simulink model "cddDcmApp"  (R2022b)
settings: Solver=FixedStepDiscrete  StartTime=0.0  StopTime=10.0  FixedStep=0.02  RelTol=1e-3
undefined symbols (not defined in the file or in its folder): CCPFaultType, DcChlType, HFAChlType, IcuChannelType, MtrRoutStaEnum, P_SignalPartType, P_Std_ReturnType, PowerChlType, SrsAdChlType, VGAChlType
(part 6 of 13)

== system cddDcmApp/DataServices_DID_980A_2_Front_ventfloor_actuator_positionfront_vent_actuator_position/DataServices_DID_980A_2_Front_ventfloor_actuator_positionfront_vent_actuator_position_FreezeCurrentState_sys ==
  function : TriggerPort  TriggerType=function-call  IsSimulinkFunction=on  FunctionName=DataServices_DID_980A_2_Front_ventfloor_actuator_positionfront_vent_actuator_position_FreezeCurrentState  VariantControl=(inherit)
  Ground_1 : Ground
  Ground_2 : Ground
  Terminator_1 : Terminator
  OpStatus : ArgIn  OutDataTypeStr=Enum: Dcm_OpStatusType  ArgumentName=OpStatus
  ErrorCode : ArgOut  OutDataTypeStr=Enum: Dcm_NegativeResponseCodeType  ArgumentName=ErrorCode
  ERR : ArgOut  Port=2  OutDataTypeStr=Std_ReturnType  ArgumentName=ERR
  wires:
  OpStatus/1 -> Terminator_1/1
  Ground_1/1 -> ErrorCode/1
  Ground_2/1 -> ERR/1

== system cddDcmApp/DataServices_DID_980A_2_Front_ventfloor_actuator_positionfront_vent_actuator_position/DataServices_DID_980A_2_Front_ventfloor_actuator_positionfront_vent_actuator_position_ReadData_sys ==
  function : TriggerPort  TriggerType=function-call  IsSimulinkFunction=on  FunctionName=DataServices_DID_980A_2_Front_ventfloor_actuator_positionfront_vent_actuator_position_ReadData  VariantControl=(inherit)
  Ground_1 : Ground
  Ground_2 : Ground
  Terminator_1 : Terminator
  OpStatus : ArgIn  OutDataTypeStr=Enum: Dcm_OpStatusType  ArgumentName=OpStatus
  Data : ArgOut  OutDataTypeStr=uint8  ArgumentName=Data
  ERR : ArgOut  Port=2  OutDataTypeStr=Std_ReturnType  ArgumentName=ERR
  wires:
  OpStatus/1 -> Terminator_1/1
  Ground_1/1 -> Data/1
  Ground_2/1 -> ERR/1

== system cddDcmApp/DataServices_DID_980A_2_Front_ventfloor_actuator_positionfront_vent_actuator_position/DataServices_DID_980A_2_Front_ventfloor_actuator_positionfront_vent_actuator_position_ReturnControlToECU_sys ==
  function : TriggerPort  TriggerType=function-call  IsSimulinkFunction=on  FunctionName=DataServices_DID_980A_2_Front_ventfloor_actuator_positionfront_vent_actuator_position_ReturnControlToECU  VariantControl=(inherit)
  Ground_1 : Ground
  Ground_2 : Ground
  ErrorCode : ArgOut  OutDataTypeStr=Enum: Dcm_NegativeResponseCodeType  ArgumentName=ErrorCode
  ERR : ArgOut  Port=2  OutDataTypeStr=Std_ReturnType  ArgumentName=ERR
  wires:
  Ground_1/1 -> ErrorCode/1
  Ground_2/1 -> ERR/1

== system cddDcmApp/DataServices_DID_980A_2_Front_ventfloor_actuator_positionfront_vent_actuator_position/DataServices_DID_980A_2_Front_ventfloor_actuator_positionfront_vent_actuator_position_ShortTermAdjustment_sys ==
  function : TriggerPort  TriggerType=function-call  IsSimulinkFunction=on  FunctionName=DataServices_DID_980A_2_Front_ventfloor_actuator_positionfront_vent_actuator_position_ShortTermAdjustment  VariantControl=(inherit)
  Ground_1 : Ground
  Ground_2 : Ground
  Terminator_1 : Terminator
  Terminator_2 : Terminator
  ControlStateInfo : ArgIn  OutDataTypeStr=uint8  ArgumentName=ControlStateInfo
  OpStatus : ArgIn  Port=2  OutDataTypeStr=Enum: Dcm_OpStatusType  ArgumentName=OpStatus
  ErrorCode : ArgOut  OutDataTypeStr=Enum: Dcm_NegativeResponseCodeType  ArgumentName=ErrorCode
  ERR : ArgOut  Port=2  OutDataTypeStr=Std_ReturnType  ArgumentName=ERR
  wires:
  ControlStateInfo/1 -> Terminator_1/1
  OpStatus/1 -> Terminator_2/1
  Ground_1/1 -> ErrorCode/1
  Ground_2/1 -> ERR/1

== system cddDcmApp/DataServices_DID_980A_DID_980A_1_Deforst_actuator_position/DataServices_DID_980A_DID_980A_1_Deforst_actuator_position_FreezeCurrentState_sys ==
  function : TriggerPort  TriggerType=function-call  IsSimulinkFunction=on  FunctionName=DataServices_DID_980A_DID_980A_1_Deforst_actuator_position_FreezeCurrentState  VariantControl=(inherit)
  Ground_1 : Ground
  Ground_2 : Ground
  Terminator_1 : Terminator
  OpStatus : ArgIn  OutDataTypeStr=Enum: Dcm_OpStatusType  ArgumentName=OpStatus
  ErrorCode : ArgOut  OutDataTypeStr=Enum: Dcm_NegativeResponseCodeType  ArgumentName=ErrorCode
  ERR : ArgOut  Port=2  OutDataTypeStr=Std_ReturnType  ArgumentName=ERR
  wires:
  OpStatus/1 -> Terminator_1/1
  Ground_1/1 -> ErrorCode/1
  Ground_2/1 -> ERR/1

== system cddDcmApp/DataServices_DID_980A_DID_980A_1_Deforst_actuator_position/DataServices_DID_980A_DID_980A_1_Deforst_actuator_position_ReadData_sys ==
  function : TriggerPort  TriggerType=function-call  IsSimulinkFunction=on  FunctionName=DataServices_DID_980A_DID_980A_1_Deforst_actuator_position_ReadData  VariantControl=(inherit)
  Ground_1 : Ground
  Ground_2 : Ground
  Terminator_1 : Terminator
  OpStatus : ArgIn  OutDataTypeStr=Enum: Dcm_OpStatusType  ArgumentName=OpStatus
  Data : ArgOut  OutDataTypeStr=uint8  ArgumentName=Data
  ERR : ArgOut  Port=2  OutDataTypeStr=Std_ReturnType  ArgumentName=ERR
  wires:
  OpStatus/1 -> Terminator_1/1
  Ground_1/1 -> Data/1
  Ground_2/1 -> ERR/1

== system cddDcmApp/DataServices_DID_980A_DID_980A_1_Deforst_actuator_position/DataServices_DID_980A_DID_980A_1_Deforst_actuator_position_ReturnControlToECU_sys ==
  function : TriggerPort  TriggerType=function-call  IsSimulinkFunction=on  FunctionName=DataServices_DID_980A_DID_980A_1_Deforst_actuator_position_ReturnControlToECU  VariantControl=(inherit)
  Ground_1 : Ground
  Ground_2 : Ground
  ErrorCode : ArgOut  OutDataTypeStr=Enum: Dcm_NegativeResponseCodeType  ArgumentName=ErrorCode
  ERR : ArgOut  Port=2  OutDataTypeStr=Std_ReturnType  ArgumentName=ERR
  wires:
  Ground_1/1 -> ErrorCode/1
  Ground_2/1 -> ERR/1

== system cddDcmApp/DataServices_DID_980A_DID_980A_1_Deforst_actuator_position/DataServices_DID_980A_DID_980A_1_Deforst_actuator_position_ShortTermAdjustment_sys ==
  function : TriggerPort  TriggerType=function-call  IsSimulinkFunction=on  FunctionName=DataServices_DID_980A_DID_980A_1_Deforst_actuator_position_ShortTermAdjustment  VariantControl=(inherit)
  Ground_1 : Ground
  Ground_2 : Ground
  Terminator_1 : Terminator
  Terminator_2 : Terminator
  ControlStateInfo : ArgIn  OutDataTypeStr=uint8  ArgumentName=ControlStateInfo
  OpStatus : ArgIn  Port=2  OutDataTypeStr=Enum: Dcm_OpStatusType  ArgumentName=OpStatus
  ErrorCode : ArgOut  OutDataTypeStr=Enum: Dcm_NegativeResponseCodeType  ArgumentName=ErrorCode
  ERR : ArgOut  Port=2  OutDataTypeStr=Std_ReturnType  ArgumentName=ERR
  wires:
  ControlStateInfo/1 -> Terminator_1/1
  OpStatus/1 -> Terminator_2/1
  Ground_1/1 -> ErrorCode/1
  Ground_2/1 -> ERR/1

== system cddDcmApp/DataServices_DID_980A_DID_980A_3_Front_floor_actuator_positionposition/DataServices_DID_980A_DID_980A_3_Front_floor_actuator_positionposition_FreezeCurrentState_sys ==
  function : TriggerPort  TriggerType=function-call  IsSimulinkFunction=on  FunctionName=DataServices_DID_980A_DID_980A_3_Front_floor_actuator_positionposition_FreezeCurrentState  VariantControl=(inherit)
  Ground_1 : Ground
  Ground_2 : Ground
  Terminator_1 : Terminator
  OpStatus : ArgIn  OutDataTypeStr=Enum: Dcm_OpStatusType  ArgumentName=OpStatus
  ErrorCode : ArgOut  OutDataTypeStr=Enum: Dcm_NegativeResponseCodeType  ArgumentName=ErrorCode
  ERR : ArgOut  Port=2  OutDataTypeStr=Std_ReturnType  ArgumentName=ERR
  wires:
  OpStatus/1 -> Terminator_1/1
  Ground_1/1 -> ErrorCode/1
  Ground_2/1 -> ERR/1

== system cddDcmApp/DataServices_DID_980A_DID_980A_3_Front_floor_actuator_positionposition/DataServices_DID_980A_DID_980A_3_Front_floor_actuator_positionposition_ReadData_sys ==
  function : TriggerPort  TriggerType=function-call  IsSimulinkFunction=on  FunctionName=DataServices_DID_980A_DID_980A_3_Front_floor_actuator_positionposition_ReadData  VariantControl=(inherit)
  Ground_1 : Ground
  Ground_2 : Ground
  Terminator_1 : Terminator
  OpStatus : ArgIn  OutDataTypeStr=Enum: Dcm_OpStatusType  ArgumentName=OpStatus
  Data : ArgOut  OutDataTypeStr=uint8  ArgumentName=Data
  ERR : ArgOut  Port=2  OutDataTypeStr=Std_ReturnType  ArgumentName=ERR
  wires:
  OpStatus/1 -> Terminator_1/1
  Ground_1/1 -> Data/1
  Ground_2/1 -> ERR/1

== system cddDcmApp/DataServices_DID_980A_DID_980A_3_Front_floor_actuator_positionposition/DataServices_DID_980A_DID_980A_3_Front_floor_actuator_positionposition_ReturnControlToECU_sys ==
  function : TriggerPort  TriggerType=function-call  IsSimulinkFunction=on  FunctionName=DataServices_DID_980A_DID_980A_3_Front_floor_actuator_positionposition_ReturnControlToECU  VariantControl=(inherit)
  Ground_1 : Ground
  Ground_2 : Ground
  ErrorCode : ArgOut  OutDataTypeStr=Enum: Dcm_NegativeResponseCodeType  ArgumentName=ErrorCode
  ERR : ArgOut  Port=2  OutDataTypeStr=Std_ReturnType  ArgumentName=ERR
  wires:
  Ground_1/1 -> ErrorCode/1
  Ground_2/1 -> ERR/1

== system cddDcmApp/DataServices_DID_980A_DID_980A_3_Front_floor_actuator_positionposition/DataServices_DID_980A_DID_980A_3_Front_floor_actuator_positionposition_ShortTermAdjustment_sys ==
  function : TriggerPort  TriggerType=function-call  IsSimulinkFunction=on  FunctionName=DataServices_DID_980A_DID_980A_3_Front_floor_actuator_positionposition_ShortTermAdjustment  VariantControl=(inherit)
  Ground_1 : Ground
  Ground_2 : Ground
  Terminator_1 : Terminator
  Terminator_2 : Terminator
  ControlStateInfo : ArgIn  OutDataTypeStr=uint8  ArgumentName=ControlStateInfo
  OpStatus : ArgIn  Port=2  OutDataTypeStr=Enum: Dcm_OpStatusType  ArgumentName=OpStatus
  ErrorCode : ArgOut  OutDataTypeStr=Enum: Dcm_NegativeResponseCodeType  ArgumentName=ErrorCode
  ERR : ArgOut  Port=2  OutDataTypeStr=Std_ReturnType  ArgumentName=ERR
  wires:
  ControlStateInfo/1 -> Terminator_1/1
  OpStatus/1 -> Terminator_2/1
  Ground_1/1 -> ErrorCode/1
  Ground_2/1 -> ERR/1

== system cddDcmApp/DataServices_DID_980A_DID_980A_4_Second_row_ventventfloor_actuator_position/DataServices_DID_980A_DID_980A_4_Second_row_ventventfloor_actuator_position_FreezeCurrentState_sys ==
  function : TriggerPort  TriggerType=function-call  IsSimulinkFunction=on  FunctionName=DataServices_DID_980A_DID_980A_4_Second_row_ventventfloor_actuator_position_FreezeCurrentState  VariantControl=(inherit)
  Ground_1 : Ground
  Ground_2 : Ground
  Terminator_1 : Terminator
  OpStatus : ArgIn  OutDataTypeStr=Enum: Dcm_OpStatusType  ArgumentName=OpStatus
  ErrorCode : ArgOut  OutDataTypeStr=Enum: Dcm_NegativeResponseCodeType  ArgumentName=ErrorCode
  ERR : ArgOut  Port=2  OutDataTypeStr=Std_ReturnType  ArgumentName=ERR
  wires:
  OpStatus/1 -> Terminator_1/1
  Ground_1/1 -> ErrorCode/1
  Ground_2/1 -> ERR/1

== system cddDcmApp/DataServices_DID_980A_DID_980A_4_Second_row_ventventfloor_actuator_position/DataServices_DID_980A_DID_980A_4_Second_row_ventventfloor_actuator_position_ReadData_sys ==
  function : TriggerPort  TriggerType=function-call  IsSimulinkFunction=on  FunctionName=DataServices_DID_980A_DID_980A_4_Second_row_ventventfloor_actuator_position_ReadData  VariantControl=(inherit)
  Ground_1 : Ground
  Ground_2 : Ground
  Terminator_1 : Terminator
  OpStatus : ArgIn  OutDataTypeStr=Enum: Dcm_OpStatusType  ArgumentName=OpStatus
  Data : ArgOut  OutDataTypeStr=uint8  ArgumentName=Data
  ERR : ArgOut  Port=2  OutDataTypeStr=Std_ReturnType  ArgumentName=ERR
  wires:
  OpStatus/1 -> Terminator_1/1
  Ground_1/1 -> Data/1
  Ground_2/1 -> ERR/1

== system cddDcmApp/DataServices_DID_980A_DID_980A_4_Second_row_ventventfloor_actuator_position/DataServices_DID_980A_DID_980A_4_Second_row_ventventfloor_actuator_position_ReturnControlToECU_sys ==
  function : TriggerPort  TriggerType=function-call  IsSimulinkFunction=on  FunctionName=DataServices_DID_980A_DID_980A_4_Second_row_ventventfloor_actuator_position_ReturnControlToECU  VariantControl=(inherit)
  Ground_1 : Ground
  Ground_2 : Ground
  ErrorCode : ArgOut  OutDataTypeStr=Enum: Dcm_NegativeResponseCodeType  ArgumentName=ErrorCode
  ERR : ArgOut  Port=2  OutDataTypeStr=Std_ReturnType  ArgumentName=ERR
  wires:
  Ground_1/1 -> ErrorCode/1
  Ground_2/1 -> ERR/1

== system cddDcmApp/DataServices_DID_980A_DID_980A_4_Second_row_ventventfloor_actuator_position/DataServices_DID_980A_DID_980A_4_Second_row_ventventfloor_actuator_position_ShortTermAdjustment_sys ==
  function : TriggerPort  TriggerType=function-call  IsSimulinkFunction=on  FunctionName=DataServices_DID_980A_DID_980A_4_Second_row_ventventfloor_actuator_position_ShortTermAdjustment  VariantControl=(inherit)
  Ground_1 : Ground
  Ground_2 : Ground
  Terminator_1 : Terminator
  Terminator_2 : Terminator
  ControlStateInfo : ArgIn  OutDataTypeStr=uint8  ArgumentName=ControlStateInfo
  OpStatus : ArgIn  Port=2  OutDataTypeStr=Enum: Dcm_OpStatusType  ArgumentName=OpStatus
  ErrorCode : ArgOut  OutDataTypeStr=Enum: Dcm_NegativeResponseCodeType  ArgumentName=ErrorCode
  ERR : ArgOut  Port=2  OutDataTypeStr=Std_ReturnType  ArgumentName=ERR
  wires:
  ControlStateInfo/1 -> Terminator_1/1
  OpStatus/1 -> Terminator_2/1
  Ground_1/1 -> ErrorCode/1
  Ground_2/1 -> ERR/1

== system cddDcmApp/DataServices_DID_980B_DID_980B_10_VGA7Passenger_Right_X_actuator_positon/DataServices_DID_980B_DID_980B_10_VGA7Passenger_Right_X_actuator_positon_FreezeCurrentState_sys ==
  function : TriggerPort  TriggerType=function-call  IsSimulinkFunction=on  FunctionName=DataServices_DID_980B_DID_980B_10_VGA7Passenger_Right_X_actuator_positon_FreezeCurrentState  VariantControl=(inherit)
  Ground_1 : Ground
  Ground_2 : Ground
  Terminator_1 : Terminator
  OpStatus : ArgIn  OutDataTypeStr=Enum: Dcm_OpStatusType  ArgumentName=OpStatus
  ErrorCode : ArgOut  OutDataTypeStr=Enum: Dcm_NegativeResponseCodeType  ArgumentName=ErrorCode
  ERR : ArgOut  Port=2  OutDataTypeStr=Std_ReturnType  ArgumentName=ERR
  wires:
  OpStatus/1 -> Terminator_1/1
  Ground_1/1 -> ErrorCode/1
  Ground_2/1 -> ERR/1

== system cddDcmApp/DataServices_DID_980B_DID_980B_10_VGA7Passenger_Right_X_actuator_positon/DataServices_DID_980B_DID_980B_10_VGA7Passenger_Right_X_actuator_positon_ReadData_sys ==
  function : TriggerPort  TriggerType=function-call  IsSimulinkFunction=on  FunctionName=DataServices_DID_980B_DID_980B_10_VGA7Passenger_Right_X_actuator_positon_ReadData  VariantControl=(inherit)
  Ground_1 : Ground
  Ground_2 : Ground
  Terminator_1 : Terminator
  OpStatus : ArgIn  OutDataTypeStr=Enum: Dcm_OpStatusType  ArgumentName=OpStatus
  Data : ArgOut  OutDataTypeStr=uint8  ArgumentName=Data
  ERR : ArgOut  Port=2  OutDataTypeStr=Std_ReturnType  ArgumentName=ERR
  wires:
  OpStatus/1 -> Terminator_1/1
  Ground_1/1 -> Data/1
  Ground_2/1 -> ERR/1

== system cddDcmApp/DataServices_DID_980B_DID_980B_10_VGA7Passenger_Right_X_actuator_positon/DataServices_DID_980B_DID_980B_10_VGA7Passenger_Right_X_actuator_positon_ReturnControlToECU_sys ==
  function : TriggerPort  TriggerType=function-call  IsSimulinkFunction=on  FunctionName=DataServices_DID_980B_DID_980B_10_VGA7Passenger_Right_X_actuator_positon_ReturnControlToECU  VariantControl=(inherit)
  Ground_1 : Ground
  Ground_2 : Ground
  ErrorCode : ArgOut  OutDataTypeStr=Enum: Dcm_NegativeResponseCodeType  ArgumentName=ErrorCode
  ERR : ArgOut  Port=2  OutDataTypeStr=Std_ReturnType  ArgumentName=ERR
  wires:
  Ground_1/1 -> ErrorCode/1
  Ground_2/1 -> ERR/1

== system cddDcmApp/DataServices_DID_980B_DID_980B_10_VGA7Passenger_Right_X_actuator_positon/DataServices_DID_980B_DID_980B_10_VGA7Passenger_Right_X_actuator_positon_ShortTermAdjustment_sys ==
  function : TriggerPort  TriggerType=function-call  IsSimulinkFunction=on  FunctionName=DataServices_DID_980B_DID_980B_10_VGA7Passenger_Right_X_actuator_positon_ShortTermAdjustment  VariantControl=(inherit)
  Ground_1 : Ground
  Ground_2 : Ground
  Terminator_1 : Terminator
  Terminator_2 : Terminator
  ControlStateInfo : ArgIn  OutDataTypeStr=uint8  ArgumentName=ControlStateInfo
  OpStatus : ArgIn  Port=2  OutDataTypeStr=Enum: Dcm_OpStatusType  ArgumentName=OpStatus
  ErrorCode : ArgOut  OutDataTypeStr=Enum: Dcm_NegativeResponseCodeType  ArgumentName=ErrorCode
  ERR : ArgOut  Port=2  OutDataTypeStr=Std_ReturnType  ArgumentName=ERR
  wires:
  ControlStateInfo/1 -> Terminator_1/1
  OpStatus/1 -> Terminator_2/1
  Ground_1/1 -> ErrorCode/1
  Ground_2/1 -> ERR/1

== system cddDcmApp/DataServices_DID_980B_DID_980B_11_VGA8Passenger_Right_Y_actuator_positon/DataServices_DID_980B_DID_980B_11_VGA8Passenger_Right_Y_actuator_positon_FreezeCurrentState_sys ==
  function : TriggerPort  TriggerType=function-call  IsSimulinkFunction=on  FunctionName=DataServices_DID_980B_DID_980B_11_VGA8Passenger_Right_Y_actuator_positon_FreezeCurrentState  VariantControl=(inherit)
  Ground_1 : Ground
  Ground_2 : Ground
  Terminator_1 : Terminator
  OpStatus : ArgIn  OutDataTypeStr=Enum: Dcm_OpStatusType  ArgumentName=OpStatus
  ErrorCode : ArgOut  OutDataTypeStr=Enum: Dcm_NegativeResponseCodeType  ArgumentName=ErrorCode
  ERR : ArgOut  Port=2  OutDataTypeStr=Std_ReturnType  ArgumentName=ERR
  wires:
  OpStatus/1 -> Terminator_1/1
  Ground_1/1 -> ErrorCode/1
  Ground_2/1 -> ERR/1

== system cddDcmApp/DataServices_DID_980B_DID_980B_11_VGA8Passenger_Right_Y_actuator_positon/DataServices_DID_980B_DID_980B_11_VGA8Passenger_Right_Y_actuator_positon_ReadData_sys ==
  function : TriggerPort  TriggerType=function-call  IsSimulinkFunction=on  FunctionName=DataServices_DID_980B_DID_980B_11_VGA8Passenger_Right_Y_actuator_positon_ReadData  VariantControl=(inherit)
  Ground_1 : Ground
  Ground_2 : Ground
  Terminator_1 : Terminator
  OpStatus : ArgIn  OutDataTypeStr=Enum: Dcm_OpStatusType  ArgumentName=OpStatus
  Data : ArgOut  OutDataTypeStr=uint8  ArgumentName=Data
  ERR : ArgOut  Port=2  OutDataTypeStr=Std_ReturnType  ArgumentName=ERR
  wires:
  OpStatus/1 -> Terminator_1/1
  Ground_1/1 -> Data/1
  Ground_2/1 -> ERR/1

== system cddDcmApp/DataServices_DID_980B_DID_980B_11_VGA8Passenger_Right_Y_actuator_positon/DataServices_DID_980B_DID_980B_11_VGA8Passenger_Right_Y_actuator_positon_ReturnControlToECU_sys ==
  function : TriggerPort  TriggerType=function-call  IsSimulinkFunction=on  FunctionName=DataServices_DID_980B_DID_980B_11_VGA8Passenger_Right_Y_actuator_positon_ReturnControlToECU  VariantControl=(inherit)
  Ground_1 : Ground
  Ground_2 : Ground
  ErrorCode : ArgOut  OutDataTypeStr=Enum: Dcm_NegativeResponseCodeType  ArgumentName=ErrorCode
  ERR : ArgOut  Port=2  OutDataTypeStr=Std_ReturnType  ArgumentName=ERR
  wires:
  Ground_1/1 -> ErrorCode/1
  Ground_2/1 -> ERR/1

== system cddDcmApp/DataServices_DID_980B_DID_980B_11_VGA8Passenger_Right_Y_actuator_positon/DataServices_DID_980B_DID_980B_11_VGA8Passenger_Right_Y_actuator_positon_ShortTermAdjustment_sys ==
  function : TriggerPort  TriggerType=function-call  IsSimulinkFunction=on  FunctionName=DataServices_DID_980B_DID_980B_11_VGA8Passenger_Right_Y_actuator_positon_ShortTermAdjustment  VariantControl=(inherit)
  Ground_1 : Ground
  Ground_2 : Ground
  Terminator_1 : Terminator
  Terminator_2 : Terminator
  ControlStateInfo : ArgIn  OutDataTypeStr=uint8  ArgumentName=ControlStateInfo
  OpStatus : ArgIn  Port=2  OutDataTypeStr=Enum: Dcm_OpStatusType  ArgumentName=OpStatus
  ErrorCode : ArgOut  OutDataTypeStr=Enum: Dcm_NegativeResponseCodeType  ArgumentName=ErrorCode
  ERR : ArgOut  Port=2  OutDataTypeStr=Std_ReturnType  ArgumentName=ERR
  wires:
  ControlStateInfo/1 -> Terminator_1/1
  OpStatus/1 -> Terminator_2/1
  Ground_1/1 -> ErrorCode/1
  Ground_2/1 -> ERR/1

== system cddDcmApp/DataServices_DID_980B_DID_980B_1_second_row_floor_actuator_position/DataServices_DID_980B_DID_980B_1_second_row_floor_actuator_position_FreezeCurrentState_sys ==
  function : TriggerPort  TriggerType=function-call  IsSimulinkFunction=on  FunctionName=DataServices_DID_980B_DID_980B_1_second_row_floor_actuator_position_FreezeCurrentState  VariantControl=(inherit)
  Ground_1 : Ground
  Ground_2 : Ground
  Terminator_1 : Terminator
  OpStatus : ArgIn  OutDataTypeStr=Enum: Dcm_OpStatusType  ArgumentName=OpStatus
  ErrorCode : ArgOut  OutDataTypeStr=Enum: Dcm_NegativeResponseCodeType  ArgumentName=ErrorCode
  ERR : ArgOut  Port=2  OutDataTypeStr=Std_ReturnType  ArgumentName=ERR
  wires:
  OpStatus/1 -> Terminator_1/1
  Ground_1/1 -> ErrorCode/1
  Ground_2/1 -> ERR/1

== system cddDcmApp/DataServices_DID_980B_DID_980B_1_second_row_floor_actuator_position/DataServices_DID_980B_DID_980B_1_second_row_floor_actuator_position_ReadData_sys ==
  function : TriggerPort  TriggerType=function-call  IsSimulinkFunction=on  FunctionName=DataServices_DID_980B_DID_980B_1_second_row_floor_actuator_position_ReadData  VariantControl=(inherit)
  Ground_1 : Ground
  Ground_2 : Ground
  Terminator_1 : Terminator
  OpStatus : ArgIn  OutDataTypeStr=Enum: Dcm_OpStatusType  ArgumentName=OpStatus
  Data : ArgOut  OutDataTypeStr=uint8  ArgumentName=Data
  ERR : ArgOut  Port=2  OutDataTypeStr=Std_ReturnType  ArgumentName=ERR
  wires:
  OpStatus/1 -> Terminator_1/1
  Ground_1/1 -> Data/1
  Ground_2/1 -> ERR/1

== system cddDcmApp/DataServices_DID_980B_DID_980B_1_second_row_floor_actuator_position/DataServices_DID_980B_DID_980B_1_second_row_floor_actuator_position_ReturnControlToECU_sys ==
  function : TriggerPort  TriggerType=function-call  IsSimulinkFunction=on  FunctionName=DataServices_DID_980B_DID_980B_1_second_row_floor_actuator_position_ReturnControlToECU  VariantControl=(inherit)
  Ground_1 : Ground
  Ground_2 : Ground
  ErrorCode : ArgOut  OutDataTypeStr=Enum: Dcm_NegativeResponseCodeType  ArgumentName=ErrorCode
  ERR : ArgOut  Port=2  OutDataTypeStr=Std_ReturnType  ArgumentName=ERR
  wires:
  Ground_1/1 -> ErrorCode/1
  Ground_2/1 -> ERR/1

== system cddDcmApp/DataServices_DID_980B_DID_980B_1_second_row_floor_actuator_position/DataServices_DID_980B_DID_980B_1_second_row_floor_actuator_position_ShortTermAdjustment_sys ==
  function : TriggerPort  TriggerType=function-call  IsSimulinkFunction=on  FunctionName=DataServices_DID_980B_DID_980B_1_second_row_floor_actuator_position_ShortTermAdjustment  VariantControl=(inherit)
  Ground_1 : Ground
  Ground_2 : Ground
  Terminator_1 : Terminator
  Terminator_2 : Terminator
  ControlStateInfo : ArgIn  OutDataTypeStr=uint8  ArgumentName=ControlStateInfo
  OpStatus : ArgIn  Port=2  OutDataTypeStr=Enum: Dcm_OpStatusType  ArgumentName=OpStatus
  ErrorCode : ArgOut  OutDataTypeStr=Enum: Dcm_NegativeResponseCodeType  ArgumentName=ErrorCode
  ERR : ArgOut  Port=2  OutDataTypeStr=Std_ReturnType  ArgumentName=ERR
  wires:
  ControlStateInfo/1 -> Terminator_1/1
  OpStatus/1 -> Terminator_2/1
  Ground_1/1 -> ErrorCode/1
  Ground_2/1 -> ERR/1

== system cddDcmApp/DataServices_DID_980B_DID_980B_2_Rec_actuator_position/DataServices_DID_980B_DID_980B_2_Rec_actuator_position_FreezeCurrentState_sys ==
  function : TriggerPort  TriggerType=function-call  IsSimulinkFunction=on  FunctionName=DataServices_DID_980B_DID_980B_2_Rec_actuator_position_FreezeCurrentState  VariantControl=(inherit)
  Ground_1 : Ground
  Ground_2 : Ground
  Terminator_1 : Terminator
  OpStatus : ArgIn  OutDataTypeStr=Enum: Dcm_OpStatusType  ArgumentName=OpStatus
  ErrorCode : ArgOut  OutDataTypeStr=Enum: Dcm_NegativeResponseCodeType  ArgumentName=ErrorCode
  ERR : ArgOut  Port=2  OutDataTypeStr=Std_ReturnType  ArgumentName=ERR
  wires:
  OpStatus/1 -> Terminator_1/1
  Ground_1/1 -> ErrorCode/1
  Ground_2/1 -> ERR/1

== system cddDcmApp/DataServices_DID_980B_DID_980B_2_Rec_actuator_position/DataServices_DID_980B_DID_980B_2_Rec_actuator_position_ReadData_sys ==
  function : TriggerPort  TriggerType=function-call  IsSimulinkFunction=on  FunctionName=DataServices_DID_980B_DID_980B_2_Rec_actuator_position_ReadData  VariantControl=(inherit)
  Ground_1 : Ground
  Ground_2 : Ground
  Terminator_1 : Terminator
  OpStatus : ArgIn  OutDataTypeStr=Enum: Dcm_OpStatusType  ArgumentName=OpStatus
  Data : ArgOut  OutDataTypeStr=uint8  ArgumentName=Data
  ERR : ArgOut  Port=2  OutDataTypeStr=Std_ReturnType  ArgumentName=ERR
  wires:
  OpStatus/1 -> Terminator_1/1
  Ground_1/1 -> Data/1
  Ground_2/1 -> ERR/1

== system cddDcmApp/DataServices_DID_980B_DID_980B_2_Rec_actuator_position/DataServices_DID_980B_DID_980B_2_Rec_actuator_position_ReturnControlToECU_sys ==
  function : TriggerPort  TriggerType=function-call  IsSimulinkFunction=on  FunctionName=DataServices_DID_980B_DID_980B_2_Rec_actuator_position_ReturnControlToECU  VariantControl=(inherit)
  Ground_1 : Ground
  Ground_2 : Ground
  ErrorCode : ArgOut  OutDataTypeStr=Enum: Dcm_NegativeResponseCodeType  ArgumentName=ErrorCode
  ERR : ArgOut  Port=2  OutDataTypeStr=Std_ReturnType  ArgumentName=ERR
  wires:
  Ground_1/1 -> ErrorCode/1
  Ground_2/1 -> ERR/1

== system cddDcmApp/DataServices_DID_980B_DID_980B_2_Rec_actuator_position/DataServices_DID_980B_DID_980B_2_Rec_actuator_position_ShortTermAdjustment_sys ==
  function : TriggerPort  TriggerType=function-call  IsSimulinkFunction=on  FunctionName=DataServices_DID_980B_DID_980B_2_Rec_actuator_position_ShortTermAdjustment  VariantControl=(inherit)
  Ground_1 : Ground
  Ground_2 : Ground
  Terminator_1 : Terminator
  Terminator_2 : Terminator
  ControlStateInfo : ArgIn  OutDataTypeStr=uint8  ArgumentName=ControlStateInfo
  OpStatus : ArgIn  Port=2  OutDataTypeStr=Enum: Dcm_OpStatusType  ArgumentName=OpStatus
  ErrorCode : ArgOut  OutDataTypeStr=Enum: Dcm_NegativeResponseCodeType  ArgumentName=ErrorCode
  ERR : ArgOut  Port=2  OutDataTypeStr=Std_ReturnType  ArgumentName=ERR
  wires:
  ControlStateInfo/1 -> Terminator_1/1
  OpStatus/1 -> Terminator_2/1
  Ground_1/1 -> ErrorCode/1
  Ground_2/1 -> ERR/1

== system cddDcmApp/DataServices_DID_980B_DID_980B_3_Osa_actuator_position/DataServices_DID_980B_DID_980B_3_Osa_actuator_position_FreezeCurrentState_sys ==
  function : TriggerPort  TriggerType=function-call  IsSimulinkFunction=on  FunctionName=DataServices_DID_980B_DID_980B_3_Osa_actuator_position_FreezeCurrentState  VariantControl=(inherit)
  Ground_1 : Ground
  Ground_2 : Ground
  Terminator_1 : Terminator
  OpStatus : ArgIn  OutDataTypeStr=Enum: Dcm_OpStatusType  ArgumentName=OpStatus
  ErrorCode : ArgOut  OutDataTypeStr=Enum: Dcm_NegativeResponseCodeType  ArgumentName=ErrorCode
  ERR : ArgOut  Port=2  OutDataTypeStr=Std_ReturnType  ArgumentName=ERR
  wires:
  OpStatus/1 -> Terminator_1/1
  Ground_1/1 -> ErrorCode/1
  Ground_2/1 -> ERR/1

== system cddDcmApp/DataServices_DID_980B_DID_980B_3_Osa_actuator_position/DataServices_DID_980B_DID_980B_3_Osa_actuator_position_ReadData_sys ==
  function : TriggerPort  TriggerType=function-call  IsSimulinkFunction=on  FunctionName=DataServices_DID_980B_DID_980B_3_Osa_actuator_position_ReadData  VariantControl=(inherit)
  Ground_1 : Ground
  Ground_2 : Ground
  Terminator_1 : Terminator
  OpStatus : ArgIn  OutDataTypeStr=Enum: Dcm_OpStatusType  ArgumentName=OpStatus
  Data : ArgOut  OutDataTypeStr=uint8  ArgumentName=Data
  ERR : ArgOut  Port=2  OutDataTypeStr=Std_ReturnType  ArgumentName=ERR
  wires:
  OpStatus/1 -> Terminator_1/1
  Ground_1/1 -> Data/1
  Ground_2/1 -> ERR/1

== system cddDcmApp/DataServices_DID_980B_DID_980B_3_Osa_actuator_position/DataServices_DID_980B_DID_980B_3_Osa_actuator_position_ReturnControlToECU_sys ==
  function : TriggerPort  TriggerType=function-call  IsSimulinkFunction=on  FunctionName=DataServices_DID_980B_DID_980B_3_Osa_actuator_position_ReturnControlToECU  VariantControl=(inherit)
  Ground_1 : Ground
  Ground_2 : Ground
  ErrorCode : ArgOut  OutDataTypeStr=Enum: Dcm_NegativeResponseCodeType  ArgumentName=ErrorCode
  ERR : ArgOut  Port=2  OutDataTypeStr=Std_ReturnType  ArgumentName=ERR
  wires:
  Ground_1/1 -> ErrorCode/1
  Ground_2/1 -> ERR/1

== system cddDcmApp/DataServices_DID_980B_DID_980B_3_Osa_actuator_position/DataServices_DID_980B_DID_980B_3_Osa_actuator_position_ShortTermAdjustment_sys ==
  function : TriggerPort  TriggerType=function-call  IsSimulinkFunction=on  FunctionName=DataServices_DID_980B_DID_980B_3_Osa_actuator_position_ShortTermAdjustment  VariantControl=(inherit)
  Ground_1 : Ground
  Ground_2 : Ground
  Terminator_1 : Terminator
  Terminator_2 : Terminator
  ControlStateInfo : ArgIn  OutDataTypeStr=uint8  ArgumentName=ControlStateInfo
  OpStatus : ArgIn  Port=2  OutDataTypeStr=Enum: Dcm_OpStatusType  ArgumentName=OpStatus
  ErrorCode : ArgOut  OutDataTypeStr=Enum: Dcm_NegativeResponseCodeType  ArgumentName=ErrorCode
  ERR : ArgOut  Port=2  OutDataTypeStr=Std_ReturnType  ArgumentName=ERR
  wires:
  ControlStateInfo/1 -> Terminator_1/1
  OpStatus/1 -> Terminator_2/1
  Ground_1/1 -> ErrorCode/1
  Ground_2/1 -> ERR/1

== system cddDcmApp/DataServices_DID_980B_DID_980B_4_VGA1Driver_Left_X_actuator_positon/DataServices_DID_980B_DID_980B_4_VGA1Driver_Left_X_actuator_positon_FreezeCurrentState_sys ==
  function : TriggerPort  TriggerType=function-call  IsSimulinkFunction=on  FunctionName=DataServices_DID_980B_DID_980B_4_VGA1Driver_Left_X_actuator_positon_FreezeCurrentState  VariantControl=(inherit)
  Ground_1 : Ground
  Ground_2 : Ground
  Terminator_1 : Terminator
  OpStatus : ArgIn  OutDataTypeStr=Enum: Dcm_OpStatusType  ArgumentName=OpStatus
  ErrorCode : ArgOut  OutDataTypeStr=Enum: Dcm_NegativeResponseCodeType  ArgumentName=ErrorCode
  ERR : ArgOut  Port=2  OutDataTypeStr=Std_ReturnType  ArgumentName=ERR
  wires:
  OpStatus/1 -> Terminator_1/1
  Ground_1/1 -> ErrorCode/1
  Ground_2/1 -> ERR/1

== system cddDcmApp/DataServices_DID_980B_DID_980B_4_VGA1Driver_Left_X_actuator_positon/DataServices_DID_980B_DID_980B_4_VGA1Driver_Left_X_actuator_positon_ReadData_sys ==
  function : TriggerPort  TriggerType=function-call  IsSimulinkFunction=on  FunctionName=DataServices_DID_980B_DID_980B_4_VGA1Driver_Left_X_actuator_positon_ReadData  VariantControl=(inherit)
  Ground_1 : Ground
  Ground_2 : Ground
  Terminator_1 : Terminator
  OpStatus : ArgIn  OutDataTypeStr=Enum: Dcm_OpStatusType  ArgumentName=OpStatus
  Data : ArgOut  OutDataTypeStr=uint8  ArgumentName=Data
  ERR : ArgOut  Port=2  OutDataTypeStr=Std_ReturnType  ArgumentName=ERR
  wires:
  OpStatus/1 -> Terminator_1/1
  Ground_1/1 -> Data/1
  Ground_2/1 -> ERR/1

== system cddDcmApp/DataServices_DID_980B_DID_980B_4_VGA1Driver_Left_X_actuator_positon/DataServices_DID_980B_DID_980B_4_VGA1Driver_Left_X_actuator_positon_ReturnControlToECU_sys ==
  function : TriggerPort  TriggerType=function-call  IsSimulinkFunction=on  FunctionName=DataServices_DID_980B_DID_980B_4_VGA1Driver_Left_X_actuator_positon_ReturnControlToECU  VariantControl=(inherit)
  Ground_1 : Ground
  Ground_2 : Ground
  ErrorCode : ArgOut  OutDataTypeStr=Enum: Dcm_NegativeResponseCodeType  ArgumentName=ErrorCode
  ERR : ArgOut  Port=2  OutDataTypeStr=Std_ReturnType  ArgumentName=ERR
  wires:
  Ground_1/1 -> ErrorCode/1
  Ground_2/1 -> ERR/1

== system cddDcmApp/DataServices_DID_980B_DID_980B_4_VGA1Driver_Left_X_actuator_positon/DataServices_DID_980B_DID_980B_4_VGA1Driver_Left_X_actuator_positon_ShortTermAdjustment_sys ==
  function : TriggerPort  TriggerType=function-call  IsSimulinkFunction=on  FunctionName=DataServices_DID_980B_DID_980B_4_VGA1Driver_Left_X_actuator_positon_ShortTermAdjustment  VariantControl=(inherit)
  Ground_1 : Ground
  Ground_2 : Ground
  Terminator_1 : Terminator
  Terminator_2 : Terminator
  ControlStateInfo : ArgIn  OutDataTypeStr=uint8  ArgumentName=ControlStateInfo
  OpStatus : ArgIn  Port=2  OutDataTypeStr=Enum: Dcm_OpStatusType  ArgumentName=OpStatus
  ErrorCode : ArgOut  OutDataTypeStr=Enum: Dcm_NegativeResponseCodeType  ArgumentName=ErrorCode
  ERR : ArgOut  Port=2  OutDataTypeStr=Std_ReturnType  ArgumentName=ERR
  wires:
  ControlStateInfo/1 -> Terminator_1/1
  OpStatus/1 -> Terminator_2/1
  Ground_1/1 -> ErrorCode/1
  Ground_2/1 -> ERR/1

== system cddDcmApp/DataServices_DID_980B_DID_980B_5_VGA2Driver_Left_Y_actuator_positon/DataServices_DID_980B_DID_980B_5_VGA2Driver_Left_Y_actuator_positon_FreezeCurrentState_sys ==
  function : TriggerPort  TriggerType=function-call  IsSimulinkFunction=on  FunctionName=DataServices_DID_980B_DID_980B_5_VGA2Driver_Left_Y_actuator_positon_FreezeCurrentState  VariantControl=(inherit)
  Ground_1 : Ground
  Ground_2 : Ground
  Terminator_1 : Terminator
  OpStatus : ArgIn  OutDataTypeStr=Enum: Dcm_OpStatusType  ArgumentName=OpStatus
  ErrorCode : ArgOut  OutDataTypeStr=Enum: Dcm_NegativeResponseCodeType  ArgumentName=ErrorCode
  ERR : ArgOut  Port=2  OutDataTypeStr=Std_ReturnType  ArgumentName=ERR
  wires:
  OpStatus/1 -> Terminator_1/1
  Ground_1/1 -> ErrorCode/1
  Ground_2/1 -> ERR/1

== system cddDcmApp/DataServices_DID_980B_DID_980B_5_VGA2Driver_Left_Y_actuator_positon/DataServices_DID_980B_DID_980B_5_VGA2Driver_Left_Y_actuator_positon_ReadData_sys ==
  function : TriggerPort  TriggerType=function-call  IsSimulinkFunction=on  FunctionName=DataServices_DID_980B_DID_980B_5_VGA2Driver_Left_Y_actuator_positon_ReadData  VariantControl=(inherit)
  Ground_1 : Ground
  Ground_2 : Ground
  Terminator_1 : Terminator
  OpStatus : ArgIn  OutDataTypeStr=Enum: Dcm_OpStatusType  ArgumentName=OpStatus
  Data : ArgOut  OutDataTypeStr=uint8  ArgumentName=Data
  ERR : ArgOut  Port=2  OutDataTypeStr=Std_ReturnType  ArgumentName=ERR
  wires:
  OpStatus/1 -> Terminator_1/1
  Ground_1/1 -> Data/1
  Ground_2/1 -> ERR/1

== system cddDcmApp/DataServices_DID_980B_DID_980B_5_VGA2Driver_Left_Y_actuator_positon/DataServices_DID_980B_DID_980B_5_VGA2Driver_Left_Y_actuator_positon_ReturnControlToECU_sys ==
  function : TriggerPort  TriggerType=function-call  IsSimulinkFunction=on  FunctionName=DataServices_DID_980B_DID_980B_5_VGA2Driver_Left_Y_actuator_positon_ReturnControlToECU  VariantControl=(inherit)
  Ground_1 : Ground
  Ground_2 : Ground
  ErrorCode : ArgOut  OutDataTypeStr=Enum: Dcm_NegativeResponseCodeType  ArgumentName=ErrorCode
  ERR : ArgOut  Port=2  OutDataTypeStr=Std_ReturnType  ArgumentName=ERR
  wires:
  Ground_1/1 -> ErrorCode/1
  Ground_2/1 -> ERR/1

== system cddDcmApp/DataServices_DID_980B_DID_980B_5_VGA2Driver_Left_Y_actuator_positon/DataServices_DID_980B_DID_980B_5_VGA2Driver_Left_Y_actuator_positon_ShortTermAdjustment_sys ==
  function : TriggerPort  TriggerType=function-call  IsSimulinkFunction=on  FunctionName=DataServices_DID_980B_DID_980B_5_VGA2Driver_Left_Y_actuator_positon_ShortTermAdjustment  VariantControl=(inherit)
  Ground_1 : Ground
  Ground_2 : Ground
  Terminator_1 : Terminator
  Terminator_2 : Terminator
  ControlStateInfo : ArgIn  OutDataTypeStr=uint8  ArgumentName=ControlStateInfo
  OpStatus : ArgIn  Port=2  OutDataTypeStr=Enum: Dcm_OpStatusType  ArgumentName=OpStatus
  ErrorCode : ArgOut  OutDataTypeStr=Enum: Dcm_NegativeResponseCodeType  ArgumentName=ErrorCode
  ERR : ArgOut  Port=2  OutDataTypeStr=Std_ReturnType  ArgumentName=ERR
  wires:
  ControlStateInfo/1 -> Terminator_1/1
  OpStatus/1 -> Terminator_2/1
  Ground_1/1 -> ErrorCode/1
  Ground_2/1 -> ERR/1

== system cddDcmApp/DataServices_DID_980B_DID_980B_6_VGA3Driver_Right_X_actuator_positon/DataServices_DID_980B_DID_980B_6_VGA3Driver_Right_X_actuator_positon_FreezeCurrentState_sys ==
  function : TriggerPort  TriggerType=function-call  IsSimulinkFunction=on  FunctionName=DataServices_DID_980B_DID_980B_6_VGA3Driver_Right_X_actuator_positon_FreezeCurrentState  VariantControl=(inherit)
  Ground_1 : Ground
  Ground_2 : Ground
  Terminator_1 : Terminator
  OpStatus : ArgIn  OutDataTypeStr=Enum: Dcm_OpStatusType  ArgumentName=OpStatus
  ErrorCode : ArgOut  OutDataTypeStr=Enum: Dcm_NegativeResponseCodeType  ArgumentName=ErrorCode
  ERR : ArgOut  Port=2  OutDataTypeStr=Std_ReturnType  ArgumentName=ERR
  wires:
  OpStatus/1 -> Terminator_1/1
  Ground_1/1 -> ErrorCode/1
  Ground_2/1 -> ERR/1

== system cddDcmApp/DataServices_DID_980B_DID_980B_6_VGA3Driver_Right_X_actuator_positon/DataServices_DID_980B_DID_980B_6_VGA3Driver_Right_X_actuator_positon_ReadData_sys ==
  function : TriggerPort  TriggerType=function-call  IsSimulinkFunction=on  FunctionName=DataServices_DID_980B_DID_980B_6_VGA3Driver_Right_X_actuator_positon_ReadData  VariantControl=(inherit)
  Ground_1 : Ground
  Ground_2 : Ground
  Terminator_1 : Terminator
  OpStatus : ArgIn  OutDataTypeStr=Enum: Dcm_OpStatusType  ArgumentName=OpStatus
  Data : ArgOut  OutDataTypeStr=uint8  ArgumentName=Data
  ERR : ArgOut  Port=2  OutDataTypeStr=Std_ReturnType  ArgumentName=ERR
  wires:
  OpStatus/1 -> Terminator_1/1
  Ground_1/1 -> Data/1
  Ground_2/1 -> ERR/1

== system cddDcmApp/DataServices_DID_980B_DID_980B_6_VGA3Driver_Right_X_actuator_positon/DataServices_DID_980B_DID_980B_6_VGA3Driver_Right_X_actuator_positon_ReturnControlToECU_sys ==
  function : TriggerPort  TriggerType=function-call  IsSimulinkFunction=on  FunctionName=DataServices_DID_980B_DID_980B_6_VGA3Driver_Right_X_actuator_positon_ReturnControlToECU  VariantControl=(inherit)
  Ground_1 : Ground
  Ground_2 : Ground
  ErrorCode : ArgOut  OutDataTypeStr=Enum: Dcm_NegativeResponseCodeType  ArgumentName=ErrorCode
  ERR : ArgOut  Port=2  OutDataTypeStr=Std_ReturnType  ArgumentName=ERR
  wires:
  Ground_1/1 -> ErrorCode/1
  Ground_2/1 -> ERR/1

== system cddDcmApp/DataServices_DID_980B_DID_980B_6_VGA3Driver_Right_X_actuator_positon/DataServices_DID_980B_DID_980B_6_VGA3Driver_Right_X_actuator_positon_ShortTermAdjustment_sys ==
  function : TriggerPort  TriggerType=function-call  IsSimulinkFunction=on  FunctionName=DataServices_DID_980B_DID_980B_6_VGA3Driver_Right_X_actuator_positon_ShortTermAdjustment  VariantControl=(inherit)
  Ground_1 : Ground
  Ground_2 : Ground
  Terminator_1 : Terminator
  Terminator_2 : Terminator
  ControlStateInfo : ArgIn  OutDataTypeStr=uint8  ArgumentName=ControlStateInfo
  OpStatus : ArgIn  Port=2  OutDataTypeStr=Enum: Dcm_OpStatusType  ArgumentName=OpStatus
  ErrorCode : ArgOut  OutDataTypeStr=Enum: Dcm_NegativeResponseCodeType  ArgumentName=ErrorCode
  ERR : ArgOut  Port=2  OutDataTypeStr=Std_ReturnType  ArgumentName=ERR
  wires:
  ControlStateInfo/1 -> Terminator_1/1
  OpStatus/1 -> Terminator_2/1
  Ground_1/1 -> ErrorCode/1
  Ground_2/1 -> ERR/1

== system cddDcmApp/DataServices_DID_980B_DID_980B_7_VGA4Driver_Right_Y_actuator_positon/DataServices_DID_980B_DID_980B_7_VGA4Driver_Right_Y_actuator_positon_FreezeCurrentState_sys ==
  function : TriggerPort  TriggerType=function-call  IsSimulinkFunction=on  FunctionName=DataServices_DID_980B_DID_980B_7_VGA4Driver_Right_Y_actuator_positon_FreezeCurrentState  VariantControl=(inherit)
  Ground_1 : Ground
  Ground_2 : Ground
  Terminator_1 : Terminator
  OpStatus : ArgIn  OutDataTypeStr=Enum: Dcm_OpStatusType  ArgumentName=OpStatus
  ErrorCode : ArgOut  OutDataTypeStr=Enum: Dcm_NegativeResponseCodeType  ArgumentName=ErrorCode
  ERR : ArgOut  Port=2  OutDataTypeStr=Std_ReturnType  ArgumentName=ERR
  wires:
  OpStatus/1 -> Terminator_1/1
  Ground_1/1 -> ErrorCode/1
  Ground_2/1 -> ERR/1

== system cddDcmApp/DataServices_DID_980B_DID_980B_7_VGA4Driver_Right_Y_actuator_positon/DataServices_DID_980B_DID_980B_7_VGA4Driver_Right_Y_actuator_positon_ReadData_sys ==
  function : TriggerPort  TriggerType=function-call  IsSimulinkFunction=on  FunctionName=DataServices_DID_980B_DID_980B_7_VGA4Driver_Right_Y_actuator_positon_ReadData  VariantControl=(inherit)
  Ground_1 : Ground
  Ground_2 : Ground
  Terminator_1 : Terminator
  OpStatus : ArgIn  OutDataTypeStr=Enum: Dcm_OpStatusType  ArgumentName=OpStatus
  Data : ArgOut  OutDataTypeStr=uint8  ArgumentName=Data
  ERR : ArgOut  Port=2  OutDataTypeStr=Std_ReturnType  ArgumentName=ERR
  wires:
  OpStatus/1 -> Terminator_1/1
  Ground_1/1 -> Data/1
  Ground_2/1 -> ERR/1

== system cddDcmApp/DataServices_DID_980B_DID_980B_7_VGA4Driver_Right_Y_actuator_positon/DataServices_DID_980B_DID_980B_7_VGA4Driver_Right_Y_actuator_positon_ReturnControlToECU_sys ==
  function : TriggerPort  TriggerType=function-call  IsSimulinkFunction=on  FunctionName=DataServices_DID_980B_DID_980B_7_VGA4Driver_Right_Y_actuator_positon_ReturnControlToECU  VariantControl=(inherit)
  Ground_1 : Ground
  Ground_2 : Ground
  ErrorCode : ArgOut  OutDataTypeStr=Enum: Dcm_NegativeResponseCodeType  ArgumentName=ErrorCode
  ERR : ArgOut  Port=2  OutDataTypeStr=Std_ReturnType  ArgumentName=ERR
  wires:
  Ground_1/1 -> ErrorCode/1
  Ground_2/1 -> ERR/1

== system cddDcmApp/DataServices_DID_980B_DID_980B_7_VGA4Driver_Right_Y_actuator_positon/DataServices_DID_980B_DID_980B_7_VGA4Driver_Right_Y_actuator_positon_ShortTermAdjustment_sys ==
  function : TriggerPort  TriggerType=function-call  IsSimulinkFunction=on  FunctionName=DataServices_DID_980B_DID_980B_7_VGA4Driver_Right_Y_actuator_positon_ShortTermAdjustment  VariantControl=(inherit)
  Ground_1 : Ground
  Ground_2 : Ground
  Terminator_1 : Terminator
  Terminator_2 : Terminator
  ControlStateInfo : ArgIn  OutDataTypeStr=uint8  ArgumentName=ControlStateInfo
  OpStatus : ArgIn  Port=2  OutDataTypeStr=Enum: Dcm_OpStatusType  ArgumentName=OpStatus
  ErrorCode : ArgOut  OutDataTypeStr=Enum: Dcm_NegativeResponseCodeType  ArgumentName=ErrorCode
  ERR : ArgOut  Port=2  OutDataTypeStr=Std_ReturnType  ArgumentName=ERR
  wires:
  ControlStateInfo/1 -> Terminator_1/1
  OpStatus/1 -> Terminator_2/1
  Ground_1/1 -> ErrorCode/1
  Ground_2/1 -> ERR/1

== system cddDcmApp/DataServices_DID_980B_DID_980B_8_VGA5Passenger_Left_X_actuator_positon/DataServices_DID_980B_DID_980B_8_VGA5Passenger_Left_X_actuator_positon_FreezeCurrentState_sys ==
  function : TriggerPort  TriggerType=function-call  IsSimulinkFunction=on  FunctionName=DataServices_DID_980B_DID_980B_8_VGA5Passenger_Left_X_actuator_positon_FreezeCurrentState  VariantControl=(inherit)
  Ground_1 : Ground
  Ground_2 : Ground
  Terminator_1 : Terminator
  OpStatus : ArgIn  OutDataTypeStr=Enum: Dcm_OpStatusType  ArgumentName=OpStatus
  ErrorCode : ArgOut  OutDataTypeStr=Enum: Dcm_NegativeResponseCodeType  ArgumentName=ErrorCode
  ERR : ArgOut  Port=2  OutDataTypeStr=Std_ReturnType  ArgumentName=ERR
  wires:
  OpStatus/1 -> Terminator_1/1
  Ground_1/1 -> ErrorCode/1
  Ground_2/1 -> ERR/1

== system cddDcmApp/DataServices_DID_980B_DID_980B_8_VGA5Passenger_Left_X_actuator_positon/DataServices_DID_980B_DID_980B_8_VGA5Passenger_Left_X_actuator_positon_ReadData_sys ==
  function : TriggerPort  TriggerType=function-call  IsSimulinkFunction=on  FunctionName=DataServices_DID_980B_DID_980B_8_VGA5Passenger_Left_X_actuator_positon_ReadData  VariantControl=(inherit)
  Ground_1 : Ground
  Ground_2 : Ground
  Terminator_1 : Terminator
  OpStatus : ArgIn  OutDataTypeStr=Enum: Dcm_OpStatusType  ArgumentName=OpStatus
  Data : ArgOut  OutDataTypeStr=uint8  ArgumentName=Data
  ERR : ArgOut  Port=2  OutDataTypeStr=Std_ReturnType  ArgumentName=ERR
  wires:
  OpStatus/1 -> Terminator_1/1
  Ground_1/1 -> Data/1
  Ground_2/1 -> ERR/1

== system cddDcmApp/DataServices_DID_980B_DID_980B_8_VGA5Passenger_Left_X_actuator_positon/DataServices_DID_980B_DID_980B_8_VGA5Passenger_Left_X_actuator_positon_ReturnControlToECU_sys ==
  function : TriggerPort  TriggerType=function-call  IsSimulinkFunction=on  FunctionName=DataServices_DID_980B_DID_980B_8_VGA5Passenger_Left_X_actuator_positon_ReturnControlToECU  VariantControl=(inherit)
  Ground_1 : Ground
  Ground_2 : Ground
  ErrorCode : ArgOut  OutDataTypeStr=Enum: Dcm_NegativeResponseCodeType  ArgumentName=ErrorCode
  ERR : ArgOut  Port=2  OutDataTypeStr=Std_ReturnType  ArgumentName=ERR
  wires:
  Ground_1/1 -> ErrorCode/1
  Ground_2/1 -> ERR/1

== system cddDcmApp/DataServices_DID_980B_DID_980B_8_VGA5Passenger_Left_X_actuator_positon/DataServices_DID_980B_DID_980B_8_VGA5Passenger_Left_X_actuator_positon_ShortTermAdjustment_sys ==
  function : TriggerPort  TriggerType=function-call  IsSimulinkFunction=on  FunctionName=DataServices_DID_980B_DID_980B_8_VGA5Passenger_Left_X_actuator_positon_ShortTermAdjustment  VariantControl=(inherit)
  Ground_1 : Ground
  Ground_2 : Ground
  Terminator_1 : Terminator
  Terminator_2 : Terminator
  ControlStateInfo : ArgIn  OutDataTypeStr=uint8  ArgumentName=ControlStateInfo
  OpStatus : ArgIn  Port=2  OutDataTypeStr=Enum: Dcm_OpStatusType  ArgumentName=OpStatus
  ErrorCode : ArgOut  OutDataTypeStr=Enum: Dcm_NegativeResponseCodeType  ArgumentName=ErrorCode
  ERR : ArgOut  Port=2  OutDataTypeStr=Std_ReturnType  ArgumentName=ERR
  wires:
  ControlStateInfo/1 -> Terminator_1/1
  OpStatus/1 -> Terminator_2/1
  Ground_1/1 -> ErrorCode/1
  Ground_2/1 -> ERR/1

== system cddDcmApp/DataServices_DID_980B_DID_980B_9_VGA6Passenger_Left_Y_actuator_positon/DataServices_DID_980B_DID_980B_9_VGA6Passenger_Left_Y_actuator_positon_FreezeCurrentState_sys ==
  function : TriggerPort  TriggerType=function-call  IsSimulinkFunction=on  FunctionName=DataServices_DID_980B_DID_980B_9_VGA6Passenger_Left_Y_actuator_positon_FreezeCurrentState  VariantControl=(inherit)
  Ground_1 : Ground
  Ground_2 : Ground
  Terminator_1 : Terminator
  OpStatus : ArgIn  OutDataTypeStr=Enum: Dcm_OpStatusType  ArgumentName=OpStatus
  ErrorCode : ArgOut  OutDataTypeStr=Enum: Dcm_NegativeResponseCodeType  ArgumentName=ErrorCode
  ERR : ArgOut  Port=2  OutDataTypeStr=Std_ReturnType  ArgumentName=ERR
  wires:
  OpStatus/1 -> Terminator_1/1
  Ground_1/1 -> ErrorCode/1
  Ground_2/1 -> ERR/1

== system cddDcmApp/DataServices_DID_980B_DID_980B_9_VGA6Passenger_Left_Y_actuator_positon/DataServices_DID_980B_DID_980B_9_VGA6Passenger_Left_Y_actuator_positon_ReadData_sys ==
  function : TriggerPort  TriggerType=function-call  IsSimulinkFunction=on  FunctionName=DataServices_DID_980B_DID_980B_9_VGA6Passenger_Left_Y_actuator_positon_ReadData  VariantControl=(inherit)
  Ground_1 : Ground
  Ground_2 : Ground
  Terminator_1 : Terminator
  OpStatus : ArgIn  OutDataTypeStr=Enum: Dcm_OpStatusType  ArgumentName=OpStatus
  Data : ArgOut  OutDataTypeStr=uint8  ArgumentName=Data
  ERR : ArgOut  Port=2  OutDataTypeStr=Std_ReturnType  ArgumentName=ERR
  wires:
  OpStatus/1 -> Terminator_1/1
  Ground_1/1 -> Data/1
  Ground_2/1 -> ERR/1

== system cddDcmApp/DataServices_DID_980B_DID_980B_9_VGA6Passenger_Left_Y_actuator_positon/DataServices_DID_980B_DID_980B_9_VGA6Passenger_Left_Y_actuator_positon_ReturnControlToECU_sys ==
  function : TriggerPort  TriggerType=function-call  IsSimulinkFunction=on  FunctionName=DataServices_DID_980B_DID_980B_9_VGA6Passenger_Left_Y_actuator_positon_ReturnControlToECU  VariantControl=(inherit)
  Ground_1 : Ground
  Ground_2 : Ground
  ErrorCode : ArgOut  OutDataTypeStr=Enum: Dcm_NegativeResponseCodeType  ArgumentName=ErrorCode
  ERR : ArgOut  Port=2  OutDataTypeStr=Std_ReturnType  ArgumentName=ERR
  wires:
  Ground_1/1 -> ErrorCode/1
  Ground_2/1 -> ERR/1

== system cddDcmApp/DataServices_DID_980B_DID_980B_9_VGA6Passenger_Left_Y_actuator_positon/DataServices_DID_980B_DID_980B_9_VGA6Passenger_Left_Y_actuator_positon_ShortTermAdjustment_sys ==
  function : TriggerPort  TriggerType=function-call  IsSimulinkFunction=on  FunctionName=DataServices_DID_980B_DID_980B_9_VGA6Passenger_Left_Y_actuator_positon_ShortTermAdjustment  VariantControl=(inherit)
  Ground_1 : Ground
  Ground_2 : Ground
  Terminator_1 : Terminator
  Terminator_2 : Terminator
  ControlStateInfo : ArgIn  OutDataTypeStr=uint8  ArgumentName=ControlStateInfo
  OpStatus : ArgIn  Port=2  OutDataTypeStr=Enum: Dcm_OpStatusType  ArgumentName=OpStatus
  ErrorCode : ArgOut  OutDataTypeStr=Enum: Dcm_NegativeResponseCodeType  ArgumentName=ErrorCode
  ERR : ArgOut  Port=2  OutDataTypeStr=Std_ReturnType  ArgumentName=ERR
  wires:
  ControlStateInfo/1 -> Terminator_1/1
  OpStatus/1 -> Terminator_2/1
  Ground_1/1 -> ErrorCode/1
  Ground_2/1 -> ERR/1

== system cddDcmApp/DataServices_DID_980E_DID_980E_1_Front_Blower_pwm/DataServices_DID_980E_DID_980E_1_Front_Blower_pwm_FreezeCurrentState_sys ==
  function : TriggerPort  TriggerType=function-call  IsSimulinkFunction=on  FunctionName=DataServices_DID_980E_DID_980E_1_Front_Blower_pwm_FreezeCurrentState  VariantControl=(inherit)
  Ground_1 : Ground
  Ground_2 : Ground
  Terminator_1 : Terminator
  OpStatus : ArgIn  OutDataTypeStr=Enum: Dcm_OpStatusType  ArgumentName=OpStatus
  ErrorCode : ArgOut  OutDataTypeStr=Enum: Dcm_NegativeResponseCodeType  ArgumentName=ErrorCode
  ERR : ArgOut  Port=2  OutDataTypeStr=Std_ReturnType  ArgumentName=ERR
  wires:
  OpStatus/1 -> Terminator_1/1
  Ground_1/1 -> ErrorCode/1
  Ground_2/1 -> ERR/1

== system cddDcmApp/DataServices_DID_980E_DID_980E_1_Front_Blower_pwm/DataServices_DID_980E_DID_980E_1_Front_Blower_pwm_ReadData_sys ==
  function : TriggerPort  TriggerType=function-call  IsSimulinkFunction=on  FunctionName=DataServices_DID_980E_DID_980E_1_Front_Blower_pwm_ReadData  VariantControl=(inherit)
  Ground_1 : Ground
  Ground_2 : Ground
  Terminator_1 : Terminator
  OpStatus : ArgIn  OutDataTypeStr=Enum: Dcm_OpStatusType  ArgumentName=OpStatus
  Data : ArgOut  OutDataTypeStr=uint8  ArgumentName=Data
  ERR : ArgOut  Port=2  OutDataTypeStr=Std_ReturnType  ArgumentName=ERR
  wires:
  OpStatus/1 -> Terminator_1/1
  Ground_1/1 -> Data/1
  Ground_2/1 -> ERR/1

== system cddDcmApp/DataServices_DID_980E_DID_980E_1_Front_Blower_pwm/DataServices_DID_980E_DID_980E_1_Front_Blower_pwm_ReturnControlToECU_sys ==
  function : TriggerPort  TriggerType=function-call  IsSimulinkFunction=on  FunctionName=DataServices_DID_980E_DID_980E_1_Front_Blower_pwm_ReturnControlToECU  VariantControl=(inherit)
  Ground_1 : Ground
  Ground_2 : Ground
  ErrorCode : ArgOut  OutDataTypeStr=Enum: Dcm_NegativeResponseCodeType  ArgumentName=ErrorCode
  ERR : ArgOut  Port=2  OutDataTypeStr=Std_ReturnType  ArgumentName=ERR
  wires:
  Ground_1/1 -> ErrorCode/1
  Ground_2/1 -> ERR/1
